simulink model "os_polyphase_synth_4096c"  (R2021a) (continued)
== system os_polyphase_synth_4096c/pfb_fir/pol1_in1_tap86 == (continued)
      | RNDSIMPLE(ACIN*B+P)
      | RNDSIMPLE(ACIN*B+P+CARRYIN)
      | RNDSIMPLE(ACIN*B+P>>17)
      | RNDSIMPLE(ACIN*B+P>>17+CARRYIN)
      | RNDSIMPLE(ACIN*B+PCIN)
      | RNDSIMPLE(ACIN*B+PCIN+CARRYIN)
      | RNDSIMPLE(ACIN*B+PCIN>>17)
      | RNDSIMPLE(ACIN*B+PCIN>>17+CARRYIN)
      | RNDSIMPLE(ACIN*BCIN)
      | RNDSIMPLE(ACIN*BCIN+C)
      | RNDSIMPLE(ACIN*BCIN+C+CARRYIN)
      | RNDSIMPLE(ACIN*BCIN+CARRYIN)
      | RNDSIMPLE(ACIN*BCIN+P)
      | RNDSIMPLE(ACIN*BCIN+P+CARRYIN)
      | RNDSIMPLE(ACIN*BCIN+P>>17)
      | RNDSIMPLE(ACIN*BCIN+P>>17+CARRYIN)
      | RNDSIMPLE(ACIN*BCIN+PCIN)
      | RNDSIMPLE(ACIN*BCIN+PCIN+CARRYIN)
      | RNDSIMPLE(ACIN*BCIN+PCIN>>17)
      | RNDSIMPLE(ACIN*BCIN+PCIN>>17+CARRYIN)
      | RNDSIMPLE(ACIN+C)
      | RNDSIMPLE(ACIN+C+CARRYIN)
      | RNDSIMPLE(ACIN+CARRYIN)
      | RNDSIMPLE(ACIN+P)
      | RNDSIMPLE(ACIN+P+CARRYIN)
      | RNDSIMPLE(ACIN+P>>17)
      | RNDSIMPLE(ACIN+P>>17+CARRYIN)
      | RNDSIMPLE(ACIN+PCIN)
      | RNDSIMPLE(ACIN+PCIN+CARRYIN)
      | RNDSIMPLE(ACIN+PCIN>>17)
      | RNDSIMPLE(ACIN+PCIN>>17+CARRYIN)
      | RNDSIMPLE(B*(A+D))
      | RNDSIMPLE(B*(A+D)+C)
      | RNDSIMPLE(B*(A+D)+C+CARRYIN)
      | RNDSIMPLE(B*(A+D)+CARRYIN)
      | RNDSIMPLE(B*(A+D)+P)
      | RNDSIMPLE(B*(A+D)+P+CARRYIN)
      | RNDSIMPLE(B*(A+D)+P>>17)
      | RNDSIMPLE(B*(A+D)+P>>17+CARRYIN)
      | RNDSIMPLE(B*(A+D)+PCIN)
      | RNDSIMPLE(B*(A+D)+PCIN+CARRYIN)
      | RNDSIMPLE(B*(A+D)+PCIN>>17)
      | RNDSIMPLE(B*(A+D)+PCIN>>17+CARRYIN)
      | RNDSIMPLE(B*(ACIN+D))
      | RNDSIMPLE(B*(ACIN+D)+C)
      | RNDSIMPLE(B*(ACIN+D)+C+CARRYIN)
      | RNDSIMPLE(B*(ACIN+D)+CARRYIN)
      | RNDSIMPLE(B*(ACIN+D)+P)
      | RNDSIMPLE(B*(ACIN+D)+P+CARRYIN)
      | RNDSIMPLE(B*(ACIN+D)+P>>17)
      | RNDSIMPLE(B*(ACIN+D)+P>>17+CARRYIN)
      | RNDSIMPLE(B*(ACIN+D)+PCIN)
      | RNDSIMPLE(B*(ACIN+D)+PCIN+CARRYIN)
      | RNDSIMPLE(B*(ACIN+D)+PCIN>>17)
      | RNDSIMPLE(B*(ACIN+D)+PCIN>>17+CARRYIN)
      | RNDSIMPLE(B*(D+A))
      | RNDSIMPLE(B*(D+A)+C)
      | RNDSIMPLE(B*(D+A)+C+CARRYIN)
      | RNDSIMPLE(B*(D+A)+CARRYIN)
      | RNDSIMPLE(B*(D+A)+P)
      | RNDSIMPLE(B*(D+A)+P+CARRYIN)
      | RNDSIMPLE(B*(D+A)+P>>17)
      | RNDSIMPLE(B*(D+A)+P>>17+CARRYIN)
      | RNDSIMPLE(B*(D+A)+PCIN)
      | RNDSIMPLE(B*(D+A)+PCIN+CARRYIN)
      | RNDSIMPLE(B*(D+A)+PCIN>>17)
      | RNDSIMPLE(B*(D+A)+PCIN>>17+CARRYIN)
      | RNDSIMPLE(B*(D+ACIN))
      | RNDSIMPLE(B*(D+ACIN)+C)
      | RNDSIMPLE(B*(D+ACIN)+C+CARRYIN)
      | RNDSIMPLE(B*(D+ACIN)+CARRYIN)
      | RNDSIMPLE(B*(D+ACIN)+P)
      | RNDSIMPLE(B*(D+ACIN)+P+CARRYIN)
      | RNDSIMPLE(B*(D+ACIN)+P>>17)
      | RNDSIMPLE(B*(D+ACIN)+P>>17+CARRYIN)
      | RNDSIMPLE(B*(D+ACIN)+PCIN)
      | RNDSIMPLE(B*(D+ACIN)+PCIN+CARRYIN)
      | RNDSIMPLE(B*(D+ACIN)+PCIN>>17)
      | RNDSIMPLE(B*(D+ACIN)+PCIN>>17+CARRYIN)
      | RNDSIMPLE(B*(D-A))
      | RNDSIMPLE(B*(D-A)+C)
      | RNDSIMPLE(B*(D-A)+C+CARRYIN)
      | RNDSIMPLE(B*(D-A)+CARRYIN)
      | RNDSIMPLE(B*(D-A)+P)
      | RNDSIMPLE(B*(D-A)+P+CARRYIN)
      | RNDSIMPLE(B*(D-A)+P>>17)
      | RNDSIMPLE(B*(D-A)+P>>17+CARRYIN)
      | RNDSIMPLE(B*(D-A)+PCIN)
      | RNDSIMPLE(B*(D-A)+PCIN+CARRYIN)
      | RNDSIMPLE(B*(D-A)+PCIN>>17)
      | RNDSIMPLE(B*(D-A)+PCIN>>17+CARRYIN)
      | RNDSIMPLE(B*(D-ACIN))
      | RNDSIMPLE(B*(D-ACIN)+C)
      | RNDSIMPLE(B*(D-ACIN)+C+CARRYIN)
      | RNDSIMPLE(B*(D-ACIN)+CARRYIN)
      | RNDSIMPLE(B*(D-ACIN)+P)
      | RNDSIMPLE(B*(D-ACIN)+P+CARRYIN)
      | RNDSIMPLE(B*(D-ACIN)+P>>17)
      | RNDSIMPLE(B*(D-ACIN)+P>>17+CARRYIN)
      | RNDSIMPLE(B*(D-ACIN)+PCIN)
      | RNDSIMPLE(B*(D-ACIN)+PCIN+CARRYIN)
      | RNDSIMPLE(B*(D-ACIN)+PCIN>>17)
      | RNDSIMPLE(B*(D-ACIN)+PCIN>>17+CARRYIN)
      | RNDSIMPLE(B*A)
      | RNDSIMPLE(B*A+C)
      | RNDSIMPLE(B*A+C+CARRYIN)
      | RNDSIMPLE(B*A+CARRYIN)
      | RNDSIMPLE(B*A+P)
      | RNDSIMPLE(B*A+P+CARRYIN)
      | RNDSIMPLE(B*A+P>>17)
      | RNDSIMPLE(B*A+P>>17+CARRYIN)
      | RNDSIMPLE(B*A+PCIN)
      | RNDSIMPLE(B*A+PCIN+CARRYIN)
      | RNDSIMPLE(B*A+PCIN>>17)
      | RNDSIMPLE(B*A+PCIN>>17+CARRYIN)
      | RNDSIMPLE(B*ACIN)
      | RNDSIMPLE(B*ACIN+C)
      | RNDSIMPLE(B*ACIN+C+CARRYIN)
      | RNDSIMPLE(B*ACIN+CARRYIN)
      | RNDSIMPLE(B*ACIN+P)
      | RNDSIMPLE(B*ACIN+P+CARRYIN)
      | RNDSIMPLE(B*ACIN+P>>17)
      | RNDSIMPLE(B*ACIN+P>>17+CARRYIN)
      | RNDSIMPLE(B*ACIN+PCIN)
      | RNDSIMPLE(B*ACIN+PCIN+CARRYIN)
      | RNDSIMPLE(B*ACIN+PCIN>>17)
      | RNDSIMPLE(B*ACIN+PCIN>>17+CARRYIN)
      | RNDSIMPLE(B*D)
      | RNDSIMPLE(B*D+C)
      | RNDSIMPLE(B*D+C+CARRYIN)
      | RNDSIMPLE(B*D+CARRYIN)
      | RNDSIMPLE(B*D+P)
      | RNDSIMPLE(B*D+P+CARRYIN)
      | RNDSIMPLE(B*D+P>>17)
      | RNDSIMPLE(B*D+P>>17+CARRYIN)
      | RNDSIMPLE(B*D+PCIN)
      | RNDSIMPLE(B*D+PCIN+CARRYIN)
      | RNDSIMPLE(B*D+PCIN>>17)
      | RNDSIMPLE(B*D+PCIN>>17+CARRYIN)
      | RNDSIMPLE(BCIN*(A+D))
      | RNDSIMPLE(BCIN*(A+D)+C)
      | RNDSIMPLE(BCIN*(A+D)+C+CARRYIN)
      | RNDSIMPLE(BCIN*(A+D)+CARRYIN)
      | RNDSIMPLE(BCIN*(A+D)+P)
      | RNDSIMPLE(BCIN*(A+D)+P+CARRYIN)
      | RNDSIMPLE(BCIN*(A+D)+P>>17)
      | RNDSIMPLE(BCIN*(A+D)+P>>17+CARRYIN)
      | RNDSIMPLE(BCIN*(A+D)+PCIN)
      | RNDSIMPLE(BCIN*(A+D)+PCIN+CARRYIN)
      | RNDSIMPLE(BCIN*(A+D)+PCIN>>17)
      | RNDSIMPLE(BCIN*(A+D)+PCIN>>17+CARRYIN)
      | RNDSIMPLE(BCIN*(ACIN+D))
      | RNDSIMPLE(BCIN*(ACIN+D)+C)
      | RNDSIMPLE(BCIN*(ACIN+D)+C+CARRYIN)
      | RNDSIMPLE(BCIN*(ACIN+D)+CARRYIN)
      | RNDSIMPLE(BCIN*(ACIN+D)+P)
      | RNDSIMPLE(BCIN*(ACIN+D)+P+CARRYIN)
      | RNDSIMPLE(BCIN*(ACIN+D)+P>>17)
      | RNDSIMPLE(BCIN*(ACIN+D)+P>>17+CARRYIN)
      | RNDSIMPLE(BCIN*(ACIN+D)+PCIN)
      | RNDSIMPLE(BCIN*(ACIN+D)+PCIN+CARRYIN)
      | RNDSIMPLE(BCIN*(ACIN+D)+PCIN>>17)
      | RNDSIMPLE(BCIN*(ACIN+D)+PCIN>>17+CARRYIN)
      | RNDSIMPLE(BCIN*(D+A))
      | RNDSIMPLE(BCIN*(D+A)+C)
      | RNDSIMPLE(BCIN*(D+A)+C+CARRYIN)
      | RNDSIMPLE(BCIN*(D+A)+CARRYIN)
      | RNDSIMPLE(BCIN*(D+A)+P)
      | RNDSIMPLE(BCIN*(D+A)+P+CARRYIN)
      | RNDSIMPLE(BCIN*(D+A)+P>>17)
      | RNDSIMPLE(BCIN*(D+A)+P>>17+CARRYIN)
      | RNDSIMPLE(BCIN*(D+A)+PCIN)
      | RNDSIMPLE(BCIN*(D+A)+PCIN+CARRYIN)
      | RNDSIMPLE(BCIN*(D+A)+PCIN>>17)
      | RNDSIMPLE(BCIN*(D+A)+PCIN>>17+CARRYIN)
      | RNDSIMPLE(BCIN*(D+ACIN))
      | RNDSIMPLE(BCIN*(D+ACIN)+C)
      | RNDSIMPLE(BCIN*(D+ACIN)+C+CARRYIN)
      | RNDSIMPLE(BCIN*(D+ACIN)+CARRYIN)
      | RNDSIMPLE(BCIN*(D+ACIN)+P)
      | RNDSIMPLE(BCIN*(D+ACIN)+P+CARRYIN)
      | RNDSIMPLE(BCIN*(D+ACIN)+P>>17)
      | RNDSIMPLE(BCIN*(D+ACIN)+P>>17+CARRYIN)
      | RNDSIMPLE(BCIN*(D+ACIN)+PCIN)
      | RNDSIMPLE(BCIN*(D+ACIN)+PCIN+CARRYIN)
      | RNDSIMPLE(BCIN*(D+ACIN)+PCIN>>17)
      | RNDSIMPLE(BCIN*(D+ACIN)+PCIN>>17+CARRYIN)
      | RNDSIMPLE(BCIN*(D-A))
      | RNDSIMPLE(BCIN*(D-A)+C)
      | RNDSIMPLE(BCIN*(D-A)+C+CARRYIN)
      | RNDSIMPLE(BCIN*(D-A)+CARRYIN)
      | RNDSIMPLE(BCIN*(D-A)+P)
      | RNDSIMPLE(BCIN*(D-A)+P+CARRYIN)
      | RNDSIMPLE(BCIN*(D-A)+P>>17)
      | RNDSIMPLE(BCIN*(D-A)+P>>17+CARRYIN)
      | RNDSIMPLE(BCIN*(D-A)+PCIN)
      | RNDSIMPLE(BCIN*(D-A)+PCIN+CARRYIN)
      | RNDSIMPLE(BCIN*(D-A)+PCIN>>17)
      | RNDSIMPLE(BCIN*(D-A)+PCIN>>17+CARRYIN)
      | RNDSIMPLE(BCIN*(D-ACIN))
      | RNDSIMPLE(BCIN*(D-ACIN)+C)
      | RNDSIMPLE(BCIN*(D-ACIN)+C+CARRYIN)
      | RNDSIMPLE(BCIN*(D-ACIN)+CARRYIN)
      | RNDSIMPLE(BCIN*(D-ACIN)+P)
      | RNDSIMPLE(BCIN*(D-ACIN)+P+CARRYIN)
      | RNDSIMPLE(BCIN*(D-ACIN)+P>>17)
      | RNDSIMPLE(BCIN*(D-ACIN)+P>>17+CARRYIN)
      | RNDSIMPLE(BCIN*(D-ACIN)+PCIN)
      | RNDSIMPLE(BCIN*(D-ACIN)+PCIN+CARRYIN)
      | RNDSIMPLE(BCIN*(D-ACIN)+PCIN>>17)
      | RNDSIMPLE(BCIN*(D-ACIN)+PCIN>>17+CARRYIN)
      | RNDSIMPLE(BCIN*A)
      | RNDSIMPLE(BCIN*A+C)
      | RNDSIMPLE(BCIN*A+C+CARRYIN)
      | RNDSIMPLE(BCIN*A+CARRYIN)
      | RNDSIMPLE(BCIN*A+P)
      | RNDSIMPLE(BCIN*A+P+CARRYIN)
      | RNDSIMPLE(BCIN*A+P>>17)
      | RNDSIMPLE(BCIN*A+P>>17+CARRYIN)
      | RNDSIMPLE(BCIN*A+PCIN)
      | RNDSIMPLE(BCIN*A+PCIN+CARRYIN)
      | RNDSIMPLE(BCIN*A+PCIN>>17)
      | RNDSIMPLE(BCIN*A+PCIN>>17+CARRYIN)
      | RNDSIMPLE(BCIN*ACIN)
      | RNDSIMPLE(BCIN*ACIN+C)
      | RNDSIMPLE(BCIN*ACIN+C+CARRYIN)
      | RNDSIMPLE(BCIN*ACIN+CARRYIN)
      | RNDSIMPLE(BCIN*ACIN+P)
      | RNDSIMPLE(BCIN*ACIN+P+CARRYIN)
      | RNDSIMPLE(BCIN*ACIN+P>>17)
      | RNDSIMPLE(BCIN*ACIN+P>>17+CARRYIN)
      | RNDSIMPLE(BCIN*ACIN+PCIN)
      | RNDSIMPLE(BCIN*ACIN+PCIN+CARRYIN)
      | RNDSIMPLE(BCIN*ACIN+PCIN>>17)
      | RNDSIMPLE(BCIN*ACIN+PCIN>>17+CARRYIN)
      | RNDSIMPLE(BCIN*D)
      | RNDSIMPLE(BCIN*D+C)
      | RNDSIMPLE(BCIN*D+C+CARRYIN)
      | RNDSIMPLE(BCIN*D+CARRYIN)
      | RNDSIMPLE(BCIN*D+P)
      | RNDSIMPLE(BCIN*D+P+CARRYIN)
      | RNDSIMPLE(BCIN*D+P>>17)
      | RNDSIMPLE(BCIN*D+P>>17+CARRYIN)
      | RNDSIMPLE(BCIN*D+PCIN)
      | RNDSIMPLE(BCIN*D+PCIN+CARRYIN)
      | RNDSIMPLE(BCIN*D+PCIN>>17)
      | RNDSIMPLE(BCIN*D+PCIN>>17+CARRYIN)
      | RNDSIMPLE(C)
      | RNDSIMPLE(C+(A+D))
      | RNDSIMPLE(C+(A+D)*(A+D))
      | RNDSIMPLE(C+(A+D)*(A+D)+CARRYIN)
      | RNDSIMPLE(C+(A+D)*B)
      | RNDSIMPLE(C+(A+D)*B+CARRYIN)
      | RNDSIMPLE(C+(A+D)*BCIN)
      | RNDSIMPLE(C+(A+D)*BCIN+CARRYIN)
      | RNDSIMPLE(C+(A+D)+CARRYIN)
      | RNDSIMPLE(C+(ACIN+D))
      | RNDSIMPLE(C+(ACIN+D)*(ACIN+D))
      | RNDSIMPLE(C+(ACIN+D)*(ACIN+D)+CARRYIN)
      | RNDSIMPLE(C+(ACIN+D)*B)
      | RNDSIMPLE(C+(ACIN+D)*B+CARRYIN)
      | RNDSIMPLE(C+(ACIN+D)*BCIN)
      | RNDSIMPLE(C+(ACIN+D)*BCIN+CARRYIN)
      | RNDSIMPLE(C+(ACIN+D)+CARRYIN)
      | RNDSIMPLE(C+(D+A))
      | RNDSIMPLE(C+(D+A)*(D+A))
      | RNDSIMPLE(C+(D+A)*(D+A)+CARRYIN)
      | RNDSIMPLE(C+(D+A)*B)
      | RNDSIMPLE(C+(D+A)*B+CARRYIN)
      | RNDSIMPLE(C+(D+A)*BCIN)
      | RNDSIMPLE(C+(D+A)*BCIN+CARRYIN)
      | RNDSIMPLE(C+(D+A)+CARRYIN)
      | RNDSIMPLE(C+(D+ACIN))
      | RNDSIMPLE(C+(D+ACIN)*(D+ACIN))
      | RNDSIMPLE(C+(D+ACIN)*(D+ACIN)+CARRYIN)
      | RNDSIMPLE(C+(D+ACIN)*B)
      | RNDSIMPLE(C+(D+ACIN)*B+CARRYIN)
      | RNDSIMPLE(C+(D+ACIN)*BCIN)
      | RNDSIMPLE(C+(D+ACIN)*BCIN+CARRYIN)
      | RNDSIMPLE(C+(D+ACIN)+CARRYIN)
      | RNDSIMPLE(C+(D-A))
      | RNDSIMPLE(C+(D-A)*(D-A))
      | RNDSIMPLE(C+(D-A)*(D-A)+CARRYIN)
      | RNDSIMPLE(C+(D-A)*B)
      | RNDSIMPLE(C+(D-A)*B+CARRYIN)
      | RNDSIMPLE(C+(D-A)*BCIN)
      | RNDSIMPLE(C+(D-A)*BCIN+CARRYIN)
      | RNDSIMPLE(C+(D-A)+CARRYIN)
      | RNDSIMPLE(C+(D-ACIN))
      | RNDSIMPLE(C+(D-ACIN)*(D-ACIN))
      | RNDSIMPLE(C+(D-ACIN)*(D-ACIN)+CARRYIN)
      | RNDSIMPLE(C+(D-ACIN)*B)
      | RNDSIMPLE(C+(D-ACIN)*B+CARRYIN)
      | RNDSIMPLE(C+(D-ACIN)*BCIN)
      | RNDSIMPLE(C+(D-ACIN)*BCIN+CARRYIN)
      | RNDSIMPLE(C+(D-ACIN)+CARRYIN)
      | RNDSIMPLE(C+A)
      | RNDSIMPLE(C+A*A)
      | RNDSIMPLE(C+A*A+CARRYIN)
      | RNDSIMPLE(C+A*B)
      | RNDSIMPLE(C+A*B+CARRYIN)
      | RNDSIMPLE(C+A*BCIN)
      | RNDSIMPLE(C+A*BCIN+CARRYIN)
      | RNDSIMPLE(C+A+CARRYIN)
      | RNDSIMPLE(C+ACIN)
      | RNDSIMPLE(C+ACIN*ACIN)
      | RNDSIMPLE(C+ACIN*ACIN+CARRYIN)
      | RNDSIMPLE(C+ACIN*B)
      | RNDSIMPLE(C+ACIN*B+CARRYIN)
      | RNDSIMPLE(C+ACIN*BCIN)
      | RNDSIMPLE(C+ACIN*BCIN+CARRYIN)
      | RNDSIMPLE(C+ACIN+CARRYIN)
      | RNDSIMPLE(C+B*(A+D))
      | RNDSIMPLE(C+B*(A+D)+CARRYIN)
      | RNDSIMPLE(C+B*(ACIN+D))
      | RNDSIMPLE(C+B*(ACIN+D)+CARRYIN)
      | RNDSIMPLE(C+B*(D+A))
      | RNDSIMPLE(C+B*(D+A)+CARRYIN)
      | RNDSIMPLE(C+B*(D+ACIN))
      | RNDSIMPLE(C+B*(D+ACIN)+CARRYIN)
      | RNDSIMPLE(C+B*(D-A))
      | RNDSIMPLE(C+B*(D-A)+CARRYIN)
      | RNDSIMPLE(C+B*(D-ACIN))
      | RNDSIMPLE(C+B*(D-ACIN)+CARRYIN)
      | RNDSIMPLE(C+B*A)
      | RNDSIMPLE(C+B*A+CARRYIN)
      | RNDSIMPLE(C+B*ACIN)
      | RNDSIMPLE(C+B*ACIN+CARRYIN)
      | RNDSIMPLE(C+B*D)
      | RNDSIMPLE(C+B*D+CARRYIN)
      | RNDSIMPLE(C+BCIN*(A+D))
      | RNDSIMPLE(C+BCIN*(A+D)+CARRYIN)
      | RNDSIMPLE(C+BCIN*(ACIN+D))
      | RNDSIMPLE(C+BCIN*(ACIN+D)+CARRYIN)
      | RNDSIMPLE(C+BCIN*(D+A))
      | RNDSIMPLE(C+BCIN*(D+A)+CARRYIN)
      | RNDSIMPLE(C+BCIN*(D+ACIN))
      | RNDSIMPLE(C+BCIN*(D+ACIN)+CARRYIN)
      | RNDSIMPLE(C+BCIN*(D-A))
      | RNDSIMPLE(C+BCIN*(D-A)+CARRYIN)
      | RNDSIMPLE(C+BCIN*(D-ACIN))
      | RNDSIMPLE(C+BCIN*(D-ACIN)+CARRYIN)
      | RNDSIMPLE(C+BCIN*A)
      | RNDSIMPLE(C+BCIN*A+CARRYIN)
      | RNDSIMPLE(C+BCIN*ACIN)
      | RNDSIMPLE(C+BCIN*ACIN+CARRYIN)
      | RNDSIMPLE(C+BCIN*D)
      | RNDSIMPLE(C+BCIN*D+CARRYIN)
      | RNDSIMPLE(C+C)
      | RNDSIMPLE(C+C+CARRYIN)
      | RNDSIMPLE(C+CARRYCASCIN)
      | RNDSIMPLE(C+CARRYIN)
      | RNDSIMPLE(C+CONCAT)
      | RNDSIMPLE(C+CONCAT+C)
      | RNDSIMPLE(C+CONCAT+C+CARRYIN)
      | RNDSIMPLE(C+CONCAT+CARRYIN)
      | RNDSIMPLE(C+D)
      | RNDSIMPLE(C+D*B)
      | RNDSIMPLE(C+D*B+CARRYIN)
      | RNDSIMPLE(C+D*BCIN)
      | RNDSIMPLE(C+D*BCIN+CARRYIN)
      | RNDSIMPLE(C+D*D)
      | RNDSIMPLE(C+D*D+CARRYIN)
      | RNDSIMPLE(C+D+CARRYIN)
      | RNDSIMPLE(C+P)
      | RNDSIMPLE(C+P+C)
      | RNDSIMPLE(C+P+C+CARRYIN)
      | RNDSIMPLE(C+P+CARRYIN)
      | RNDSIMPLE(CARRYIN)
      | RNDSIMPLE(CONCAT)
      | RNDSIMPLE(CONCAT+C)
      | RNDSIMPLE(CONCAT+C+CARRYIN)
      | RNDSIMPLE(CONCAT+CARRYCASCIN)
      | RNDSIMPLE(CONCAT+CARRYIN)
      | RNDSIMPLE(D)
      | RNDSIMPLE(D*B)
      | RNDSIMPLE(D*B+C)
      | RNDSIMPLE(D*B+C+CARRYIN)
      | RNDSIMPLE(D*B+CARRYIN)
      | RNDSIMPLE(D*B+P)
      | RNDSIMPLE(D*B+P+CARRYIN)
      | RNDSIMPLE(D*B+P>>17)
      | RNDSIMPLE(D*B+P>>17+CARRYIN)
      | RNDSIMPLE(D*B+PCIN)
      | RNDSIMPLE(D*B+PCIN+CARRYIN)
      | RNDSIMPLE(D*B+PCIN>>17)
      | RNDSIMPLE(D*B+PCIN>>17+CARRYIN)
      | RNDSIMPLE(D*BCIN)
      | RNDSIMPLE(D*BCIN+C)
      | RNDSIMPLE(D*BCIN+C+CARRYIN)
      | RNDSIMPLE(D*BCIN+CARRYIN)
      | RNDSIMPLE(D*BCIN+P)
      | RNDSIMPLE(D*BCIN+P+CARRYIN)
      | RNDSIMPLE(D*BCIN+P>>17)
      | RNDSIMPLE(D*BCIN+P>>17+CARRYIN)
      | RNDSIMPLE(D*BCIN+PCIN)
      | RNDSIMPLE(D*BCIN+PCIN+CARRYIN)
      | RNDSIMPLE(D*BCIN+PCIN>>17)
      | RNDSIMPLE(D*BCIN+PCIN>>17+CARRYIN)
      | RNDSIMPLE(D*D)
      | RNDSIMPLE(D*D+C)
      | RNDSIMPLE(D*D+C+CARRYIN)
      | RNDSIMPLE(D*D+CARRYIN)
      | RNDSIMPLE(D*D+P)
      | RNDSIMPLE(D*D+P+CARRYIN)
      | RNDSIMPLE(D*D+P>>17)
      | RNDSIMPLE(D*D+P>>17+CARRYIN)
      | RNDSIMPLE(D*D+PCIN)
      | RNDSIMPLE(D*D+PCIN+CARRYIN)
      | RNDSIMPLE(D*D+PCIN>>17)
      | RNDSIMPLE(D*D+PCIN>>17+CARRYIN)
      | RNDSIMPLE(D+C)
      | RNDSIMPLE(D+C+CARRYIN)
      | RNDSIMPLE(D+CARRYIN)
      | RNDSIMPLE(D+P)
      | RNDSIMPLE(D+P+CARRYIN)
      | RNDSIMPLE(D+P>>17)
      | RNDSIMPLE(D+P>>17+CARRYIN)
      | RNDSIMPLE(D+PCIN)
      | RNDSIMPLE(D+PCIN+CARRYIN)
      | RNDSIMPLE(D+PCIN>>17)
      | RNDSIMPLE(D+PCIN>>17+CARRYIN)
      | RNDSIMPLE(P)
      | RNDSIMPLE(P+(A+D))
      | RNDSIMPLE(P+(A+D)*(A+D))
      | RNDSIMPLE(P+(A+D)*(A+D)+CARRYIN)
      | RNDSIMPLE(P+(A+D)*B)
      | RNDSIMPLE(P+(A+D)*B+CARRYIN)
      | RNDSIMPLE(P+(A+D)*BCIN)
      | RNDSIMPLE(P+(A+D)*BCIN+CARRYIN)
      | RNDSIMPLE(P+(A+D)+CARRYIN)
      | RNDSIMPLE(P+(ACIN+D))
      | RNDSIMPLE(P+(ACIN+D)*(ACIN+D))
      | RNDSIMPLE(P+(ACIN+D)*(ACIN+D)+CARRYIN)
      | RNDSIMPLE(P+(ACIN+D)*B)
      | RNDSIMPLE(P+(ACIN+D)*B+CARRYIN)
      | RNDSIMPLE(P+(ACIN+D)*BCIN)
      | RNDSIMPLE(P+(ACIN+D)*BCIN+CARRYIN)
      | RNDSIMPLE(P+(ACIN+D)+CARRYIN)
      | RNDSIMPLE(P+(D+A))
      | RNDSIMPLE(P+(D+A)*(D+A))
      | RNDSIMPLE(P+(D+A)*(D+A)+CARRYIN)
      | RNDSIMPLE(P+(D+A)*B)
      | RNDSIMPLE(P+(D+A)*B+CARRYIN)
      | RNDSIMPLE(P+(D+A)*BCIN)
      | RNDSIMPLE(P+(D+A)*BCIN+CARRYIN)
      | RNDSIMPLE(P+(D+A)+CARRYIN)
      | RNDSIMPLE(P+(D+ACIN))
      | RNDSIMPLE(P+(D+ACIN)*(D+ACIN))
      | RNDSIMPLE(P+(D+ACIN)*(D+ACIN)+CARRYIN)
      | RNDSIMPLE(P+(D+ACIN)*B)
      | RNDSIMPLE(P+(D+ACIN)*B+CARRYIN)
      | RNDSIMPLE(P+(D+ACIN)*BCIN)
      | RNDSIMPLE(P+(D+ACIN)*BCIN+CARRYIN)
      | RNDSIMPLE(P+(D+ACIN)+CARRYIN)
      | RNDSIMPLE(P+(D-A))
      | RNDSIMPLE(P+(D-A)*(D-A))
      | RNDSIMPLE(P+(D-A)*(D-A)+CARRYIN)
      | RNDSIMPLE(P+(D-A)*B)
      | RNDSIMPLE(P+(D-A)*B+CARRYIN)
      | RNDSIMPLE(P+(D-A)*BCIN)
      | RNDSIMPLE(P+(D-A)*BCIN+CARRYIN)
      | RNDSIMPLE(P+(D-A)+CARRYIN)
      | RNDSIMPLE(P+(D-ACIN))
      | RNDSIMPLE(P+(D-ACIN)*(D-ACIN))
      | RNDSIMPLE(P+(D-ACIN)*(D-ACIN)+CARRYIN)
      | RNDSIMPLE(P+(D-ACIN)*B)
      | RNDSIMPLE(P+(D-ACIN)*B+CARRYIN)
      | RNDSIMPLE(P+(D-ACIN)*BCIN)
      | RNDSIMPLE(P+(D-ACIN)*BCIN+CARRYIN)
      | RNDSIMPLE(P+(D-ACIN)+CARRYIN)
      | RNDSIMPLE(P+A)
      | RNDSIMPLE(P+A*A)
      | RNDSIMPLE(P+A*A+CARRYIN)
      | RNDSIMPLE(P+A*B)
      | RNDSIMPLE(P+A*B+CARRYIN)
      | RNDSIMPLE(P+A*BCIN)
      | RNDSIMPLE(P+A*BCIN+CARRYIN)
      | RNDSIMPLE(P+A+CARRYIN)
      | RNDSIMPLE(P+ACIN)
      | RNDSIMPLE(P+ACIN*ACIN)
      | RNDSIMPLE(P+ACIN*ACIN+CARRYIN)
      | RNDSIMPLE(P+ACIN*B)
      | RNDSIMPLE(P+ACIN*B+CARRYIN)
      | RNDSIMPLE(P+ACIN*BCIN)
      | RNDSIMPLE(P+ACIN*BCIN+CARRYIN)
      | RNDSIMPLE(P+ACIN+CARRYIN)
      | RNDSIMPLE(P+B*(A+D))
      | RNDSIMPLE(P+B*(A+D)+CARRYIN)
      | RNDSIMPLE(P+B*(ACIN+D))
      | RNDSIMPLE(P+B*(ACIN+D)+CARRYIN)
      | RNDSIMPLE(P+B*(D+A))
      | RNDSIMPLE(P+B*(D+A)+CARRYIN)
      | RNDSIMPLE(P+B*(D+ACIN))
      | RNDSIMPLE(P+B*(D+ACIN)+CARRYIN)
      | RNDSIMPLE(P+B*(D-A))
      | RNDSIMPLE(P+B*(D-A)+CARRYIN)
      | RNDSIMPLE(P+B*(D-ACIN))
      | RNDSIMPLE(P+B*(D-ACIN)+CARRYIN)
      | RNDSIMPLE(P+B*A)
      | RNDSIMPLE(P+B*A+CARRYIN)
      | RNDSIMPLE(P+B*ACIN)
      | RNDSIMPLE(P+B*ACIN+CARRYIN)
      | RNDSIMPLE(P+B*D)
      | RNDSIMPLE(P+B*D+CARRYIN)
      | RNDSIMPLE(P+BCIN*(A+D))
      | RNDSIMPLE(P+BCIN*(A+D)+CARRYIN)
      | RNDSIMPLE(P+BCIN*(ACIN+D))
      | RNDSIMPLE(P+BCIN*(ACIN+D)+CARRYIN)
      | RNDSIMPLE(P+BCIN*(D+A))
      | RNDSIMPLE(P+BCIN*(D+A)+CARRYIN)
      | RNDSIMPLE(P+BCIN*(D+ACIN))
      | RNDSIMPLE(P+BCIN*(D+ACIN)+CARRYIN)
      | RNDSIMPLE(P+BCIN*(D-A))
      | RNDSIMPLE(P+BCIN*(D-A)+CARRYIN)
      | RNDSIMPLE(P+BCIN*(D-ACIN))
      | RNDSIMPLE(P+BCIN*(D-ACIN)+CARRYIN)
      | RNDSIMPLE(P+BCIN*A)
      | RNDSIMPLE(P+BCIN*A+CARRYIN)
      | RNDSIMPLE(P+BCIN*ACIN)
      | RNDSIMPLE(P+BCIN*ACIN+CARRYIN)
      | RNDSIMPLE(P+BCIN*D)
      | RNDSIMPLE(P+BCIN*D+CARRYIN)
      | RNDSIMPLE(P+C)
      | RNDSIMPLE(P+C+CARRYIN)
      | RNDSIMPLE(P+CARRYCASCIN)
      | RNDSIMPLE(P+CARRYIN)
      | RNDSIMPLE(P+CONCAT)
      | RNDSIMPLE(P+CONCAT+C)
      | RNDSIMPLE(P+CONCAT+C+CARRYIN)
      | RNDSIMPLE(P+CONCAT+CARRYIN)
      | RNDSIMPLE(P+D)
      | RNDSIMPLE(P+D*B)
      | RNDSIMPLE(P+D*B+CARRYIN)
      | RNDSIMPLE(P+D*BCIN)
      | RNDSIMPLE(P+D*BCIN+CARRYIN)
      | RNDSIMPLE(P+D*D)
      | RNDSIMPLE(P+D*D+CARRYIN)
      | RNDSIMPLE(P+D+CARRYIN)
      | RNDSIMPLE(P+P)
      | RNDSIMPLE(P+P+C)
      | RNDSIMPLE(P+P+C+CARRYIN)
      | RNDSIMPLE(P+P+CARRYIN)
      | RNDSIMPLE(P>>17)
      | RNDSIMPLE(P>>17+(A+D))
      | RNDSIMPLE(P>>17+(A+D)*(A+D))
      | RNDSIMPLE(P>>17+(A+D)*(A+D)+CARRYIN)
      | RNDSIMPLE(P>>17+(A+D)*B)
      | RNDSIMPLE(P>>17+(A+D)*B+CARRYIN)
      | RNDSIMPLE(P>>17+(A+D)*BCIN)
      | RNDSIMPLE(P>>17+(A+D)*BCIN+CARRYIN)
      | RNDSIMPLE(P>>17+(A+D)+CARRYIN)
      | RNDSIMPLE(P>>17+(ACIN+D))
      | RNDSIMPLE(P>>17+(ACIN+D)*(ACIN+D))
      | RNDSIMPLE(P>>17+(ACIN+D)*(ACIN+D)+CARRYIN)
      | RNDSIMPLE(P>>17+(ACIN+D)*B)
      | RNDSIMPLE(P>>17+(ACIN+D)*B+CARRYIN)
      | RNDSIMPLE(P>>17+(ACIN+D)*BCIN)
      | RNDSIMPLE(P>>17+(ACIN+D)*BCIN+CARRYIN)
      | RNDSIMPLE(P>>17+(ACIN+D)+CARRYIN)
      | RNDSIMPLE(P>>17+(D+A))
      | RNDSIMPLE(P>>17+(D+A)*(D+A))
      | RNDSIMPLE(P>>17+(D+A)*(D+A)+CARRYIN)
      | RNDSIMPLE(P>>17+(D+A)*B)
      | RNDSIMPLE(P>>17+(D+A)*B+CARRYIN)
      | RNDSIMPLE(P>>17+(D+A)*BCIN)
      | RNDSIMPLE(P>>17+(D+A)*BCIN+CARRYIN)
      | RNDSIMPLE(P>>17+(D+A)+CARRYIN)
      | RNDSIMPLE(P>>17+(D+ACIN))
      | RNDSIMPLE(P>>17+(D+ACIN)*(D+ACIN))
      | RNDSIMPLE(P>>17+(D+ACIN)*(D+ACIN)+CARRYIN)
      | RNDSIMPLE(P>>17+(D+ACIN)*B)
      | RNDSIMPLE(P>>17+(D+ACIN)*B+CARRYIN)
      | RNDSIMPLE(P>>17+(D+ACIN)*BCIN)
      | RNDSIMPLE(P>>17+(D+ACIN)*BCIN+CARRYIN)
      | RNDSIMPLE(P>>17+(D+ACIN)+CARRYIN)
      | RNDSIMPLE(P>>17+(D-A))
      | RNDSIMPLE(P>>17+(D-A)*(D-A))
      | RNDSIMPLE(P>>17+(D-A)*(D-A)+CARRYIN)
      | RNDSIMPLE(P>>17+(D-A)*B)
      | RNDSIMPLE(P>>17+(D-A)*B+CARRYIN)
      | RNDSIMPLE(P>>17+(D-A)*BCIN)
      | RNDSIMPLE(P>>17+(D-A)*BCIN+CARRYIN)
      | RNDSIMPLE(P>>17+(D-A)+CARRYIN)
      | RNDSIMPLE(P>>17+(D-ACIN))
      | RNDSIMPLE(P>>17+(D-ACIN)*(D-ACIN))
      | RNDSIMPLE(P>>17+(D-ACIN)*(D-ACIN)+CARRYIN)
      | RNDSIMPLE(P>>17+(D-ACIN)*B)
      | RNDSIMPLE(P>>17+(D-ACIN)*B+CARRYIN)
      | RNDSIMPLE(P>>17+(D-ACIN)*BCIN)
      | RNDSIMPLE(P>>17+(D-ACIN)*BCIN+CARRYIN)
      | RNDSIMPLE(P>>17+(D-ACIN)+CARRYIN)
      | RNDSIMPLE(P>>17+A)
      | RNDSIMPLE(P>>17+A*A)
      | RNDSIMPLE(P>>17+A*A+CARRYIN)
      | RNDSIMPLE(P>>17+A*B)
      | RNDSIMPLE(P>>17+A*B+CARRYIN)
      | RNDSIMPLE(P>>17+A*BCIN)
      | RNDSIMPLE(P>>17+A*BCIN+CARRYIN)
      | RNDSIMPLE(P>>17+A+CARRYIN)
      | RNDSIMPLE(P>>17+ACIN)
      | RNDSIMPLE(P>>17+ACIN*ACIN)
      | RNDSIMPLE(P>>17+ACIN*ACIN+CARRYIN)
      | RNDSIMPLE(P>>17+ACIN*B)
      | RNDSIMPLE(P>>17+ACIN*B+CARRYIN)
      | RNDSIMPLE(P>>17+ACIN*BCIN)
      | RNDSIMPLE(P>>17+ACIN*BCIN+CARRYIN)
      | RNDSIMPLE(P>>17+ACIN+CARRYIN)
      | RNDSIMPLE(P>>17+B*(A+D))
      | RNDSIMPLE(P>>17+B*(A+D)+CARRYIN)
      | RNDSIMPLE(P>>17+B*(ACIN+D))
      | RNDSIMPLE(P>>17+B*(ACIN+D)+CARRYIN)
      | RNDSIMPLE(P>>17+B*(D+A))
      | RNDSIMPLE(P>>17+B*(D+A)+CARRYIN)
      | RNDSIMPLE(P>>17+B*(D+ACIN))
      | RNDSIMPLE(P>>17+B*(D+ACIN)+CARRYIN)
      | RNDSIMPLE(P>>17+B*(D-A))
      | RNDSIMPLE(P>>17+B*(D-A)+CARRYIN)
      | RNDSIMPLE(P>>17+B*(D-ACIN))
      | RNDSIMPLE(P>>17+B*(D-ACIN)+CARRYIN)
      | RNDSIMPLE(P>>17+B*A)
      | RNDSIMPLE(P>>17+B*A+CARRYIN)
      | RNDSIMPLE(P>>17+B*ACIN)
      | RNDSIMPLE(P>>17+B*ACIN+CARRYIN)
      | RNDSIMPLE(P>>17+B*D)
      | RNDSIMPLE(P>>17+B*D+CARRYIN)
      | RNDSIMPLE(P>>17+BCIN*(A+D))
      | RNDSIMPLE(P>>17+BCIN*(A+D)+CARRYIN)
      | RNDSIMPLE(P>>17+BCIN*(ACIN+D))
      | RNDSIMPLE(P>>17+BCIN*(ACIN+D)+CARRYIN)
      | RNDSIMPLE(P>>17+BCIN*(D+A))
      | RNDSIMPLE(P>>17+BCIN*(D+A)+CARRYIN)
      | RNDSIMPLE(P>>17+BCIN*(D+ACIN))
      | RNDSIMPLE(P>>17+BCIN*(D+ACIN)+CARRYIN)
      | RNDSIMPLE(P>>17+BCIN*(D-A))
      | RNDSIMPLE(P>>17+BCIN*(D-A)+CARRYIN)
      | RNDSIMPLE(P>>17+BCIN*(D-ACIN))
      | RNDSIMPLE(P>>17+BCIN*(D-ACIN)+CARRYIN)
      | RNDSIMPLE(P>>17+BCIN*A)
      | RNDSIMPLE(P>>17+BCIN*A+CARRYIN)
      | RNDSIMPLE(P>>17+BCIN*ACIN)
      | RNDSIMPLE(P>>17+BCIN*ACIN+CARRYIN)
      | RNDSIMPLE(P>>17+BCIN*D)
      | RNDSIMPLE(P>>17+BCIN*D+CARRYIN)
      | RNDSIMPLE(P>>17+C)
      | RNDSIMPLE(P>>17+C+CARRYIN)
      | RNDSIMPLE(P>>17+CARRYIN)
      | RNDSIMPLE(P>>17+CONCAT)
      | RNDSIMPLE(P>>17+CONCAT+C)
      | RNDSIMPLE(P>>17+CONCAT+C+CARRYIN)
      | RNDSIMPLE(P>>17+CONCAT+CARRYIN)
      | RNDSIMPLE(P>>17+D)
      | RNDSIMPLE(P>>17+D*B)
      | RNDSIMPLE(P>>17+D*B+CARRYIN)
      | RNDSIMPLE(P>>17+D*BCIN)
      | RNDSIMPLE(P>>17+D*BCIN+CARRYIN)
      | RNDSIMPLE(P>>17+D*D)
      | RNDSIMPLE(P>>17+D*D+CARRYIN)
      | RNDSIMPLE(P>>17+D+CARRYIN)
      | RNDSIMPLE(P>>17+P)
      | RNDSIMPLE(P>>17+P+C)
      | RNDSIMPLE(P>>17+P+C+CARRYIN)
      | RNDSIMPLE(P>>17+P+CARRYIN)
      | RNDSIMPLE(PCIN)
      | RNDSIMPLE(PCIN+(A+D))
      | RNDSIMPLE(PCIN+(A+D)*(A+D))
      | RNDSIMPLE(PCIN+(A+D)*(A+D)+CARRYIN)
      | RNDSIMPLE(PCIN+(A+D)*B)
      | RNDSIMPLE(PCIN+(A+D)*B+CARRYIN)
      | RNDSIMPLE(PCIN+(A+D)*BCIN)
      | RNDSIMPLE(PCIN+(A+D)*BCIN+CARRYIN)
      | RNDSIMPLE(PCIN+(A+D)+CARRYIN)
      | RNDSIMPLE(PCIN+(ACIN+D))
      | RNDSIMPLE(PCIN+(ACIN+D)*(ACIN+D))
      | RNDSIMPLE(PCIN+(ACIN+D)*(ACIN+D)+CARRYIN)
      | RNDSIMPLE(PCIN+(ACIN+D)*B)
      | RNDSIMPLE(PCIN+(ACIN+D)*B+CARRYIN)
      | RNDSIMPLE(PCIN+(ACIN+D)*BCIN)
      | RNDSIMPLE(PCIN+(ACIN+D)*BCIN+CARRYIN)
      | RNDSIMPLE(PCIN+(ACIN+D)+CARRYIN)
      | RNDSIMPLE(PCIN+(D+A))
      | RNDSIMPLE(PCIN+(D+A)*(D+A))
      | RNDSIMPLE(PCIN+(D+A)*(D+A)+CARRYIN)
      | RNDSIMPLE(PCIN+(D+A)*B)
      | RNDSIMPLE(PCIN+(D+A)*B+CARRYIN)
      | RNDSIMPLE(PCIN+(D+A)*BCIN)
      | RNDSIMPLE(PCIN+(D+A)*BCIN+CARRYIN)
      | RNDSIMPLE(PCIN+(D+A)+CARRYIN)
      | RNDSIMPLE(PCIN+(D+ACIN))
      | RNDSIMPLE(PCIN+(D+ACIN)*(D+ACIN))
      | RNDSIMPLE(PCIN+(D+ACIN)*(D+ACIN)+CARRYIN)
      | RNDSIMPLE(PCIN+(D+ACIN)*B)
      | RNDSIMPLE(PCIN+(D+ACIN)*B+CARRYIN)
      | RNDSIMPLE(PCIN+(D+ACIN)*BCIN)
      | RNDSIMPLE(PCIN+(D+ACIN)*BCIN+CARRYIN)
      | RNDSIMPLE(PCIN+(D+ACIN)+CARRYIN)
      | RNDSIMPLE(PCIN+(D-A))
      | RNDSIMPLE(PCIN+(D-A)*(D-A))
      | RNDSIMPLE(PCIN+(D-A)*(D-A)+CARRYIN)
      | RNDSIMPLE(PCIN+(D-A)*B)
      | RNDSIMPLE(PCIN+(D-A)*B+CARRYIN)
      | RNDSIMPLE(PCIN+(D-A)*BCIN)
      | RNDSIMPLE(PCIN+(D-A)*BCIN+CARRYIN)
      | RNDSIMPLE(PCIN+(D-A)+CARRYIN)
      | RNDSIMPLE(PCIN+(D-ACIN))
      | RNDSIMPLE(PCIN+(D-ACIN)*(D-ACIN))
      | RNDSIMPLE(PCIN+(D-ACIN)*(D-ACIN)+CARRYIN)
      | RNDSIMPLE(PCIN+(D-ACIN)*B)
      | RNDSIMPLE(PCIN+(D-ACIN)*B+CARRYIN)
      | RNDSIMPLE(PCIN+(D-ACIN)*BCIN)
      | RNDSIMPLE(PCIN+(D-ACIN)*BCIN+CARRYIN)
      | RNDSIMPLE(PCIN+(D-ACIN)+CARRYIN)
      | RNDSIMPLE(PCIN+A)
      | RNDSIMPLE(PCIN+A*A)
      | RNDSIMPLE(PCIN+A*A+CARRYIN)
      | RNDSIMPLE(PCIN+A*B)
      | RNDSIMPLE(PCIN+A*B+CARRYIN)
      | RNDSIMPLE(PCIN+A*BCIN)
      | RNDSIMPLE(PCIN+A*BCIN+CARRYIN)
      | RNDSIMPLE(PCIN+A+CARRYIN)
      | RNDSIMPLE(PCIN+ACIN)
      | RNDSIMPLE(PCIN+ACIN*ACIN)
      | RNDSIMPLE(PCIN+ACIN*ACIN+CARRYIN)
      | RNDSIMPLE(PCIN+ACIN*B)
      | RNDSIMPLE(PCIN+ACIN*B+CARRYIN)
      | RNDSIMPLE(PCIN+ACIN*BCIN)
      | RNDSIMPLE(PCIN+ACIN*BCIN+CARRYIN)
      | RNDSIMPLE(PCIN+ACIN+CARRYIN)
      | RNDSIMPLE(PCIN+B*(A+D))
      | RNDSIMPLE(PCIN+B*(A+D)+CARRYIN)
      | RNDSIMPLE(PCIN+B*(ACIN+D))
      | RNDSIMPLE(PCIN+B*(ACIN+D)+CARRYIN)
      | RNDSIMPLE(PCIN+B*(D+A))
      | RNDSIMPLE(PCIN+B*(D+A)+CARRYIN)
      | RNDSIMPLE(PCIN+B*(D+ACIN))
      | RNDSIMPLE(PCIN+B*(D+ACIN)+CARRYIN)
      | RNDSIMPLE(PCIN+B*(D-A))
      | RNDSIMPLE(PCIN+B*(D-A)+CARRYIN)
      | RNDSIMPLE(PCIN+B*(D-ACIN))
      | RNDSIMPLE(PCIN+B*(D-ACIN)+CARRYIN)
      | RNDSIMPLE(PCIN+B*A)
      | RNDSIMPLE(PCIN+B*A+CARRYIN)
      | RNDSIMPLE(PCIN+B*ACIN)
      | RNDSIMPLE(PCIN+B*ACIN+CARRYIN)
      | RNDSIMPLE(PCIN+B*D)
      | RNDSIMPLE(PCIN+B*D+CARRYIN)
      | RNDSIMPLE(PCIN+BCIN*(A+D))
      | RNDSIMPLE(PCIN+BCIN*(A+D)+CARRYIN)
      | RNDSIMPLE(PCIN+BCIN*(ACIN+D))
      | RNDSIMPLE(PCIN+BCIN*(ACIN+D)+CARRYIN)
      | RNDSIMPLE(PCIN+BCIN*(D+A))
      | RNDSIMPLE(PCIN+BCIN*(D+A)+CARRYIN)
      | RNDSIMPLE(PCIN+BCIN*(D+ACIN))
      | RNDSIMPLE(PCIN+BCIN*(D+ACIN)+CARRYIN)
      | RNDSIMPLE(PCIN+BCIN*(D-A))
      | RNDSIMPLE(PCIN+BCIN*(D-A)+CARRYIN)
      | RNDSIMPLE(PCIN+BCIN*(D-ACIN))
      | RNDSIMPLE(PCIN+BCIN*(D-ACIN)+CARRYIN)
      | RNDSIMPLE(PCIN+BCIN*A)
      | RNDSIMPLE(PCIN+BCIN*A+CARRYIN)
      | RNDSIMPLE(PCIN+BCIN*ACIN)
      | RNDSIMPLE(PCIN+BCIN*ACIN+CARRYIN)
      | RNDSIMPLE(PCIN+BCIN*D)
      | RNDSIMPLE(PCIN+BCIN*D+CARRYIN)
      | RNDSIMPLE(PCIN+C)
      | RNDSIMPLE(PCIN+C+CARRYIN)
      | RNDSIMPLE(PCIN+CARRYIN)
      | RNDSIMPLE(PCIN+CONCAT)
      | RNDSIMPLE(PCIN+CONCAT+C)
      | RNDSIMPLE(PCIN+CONCAT+C+CARRYIN)
      | RNDSIMPLE(PCIN+CONCAT+CARRYIN)
      | RNDSIMPLE(PCIN+D)
      | RNDSIMPLE(PCIN+D*B)
      | RNDSIMPLE(PCIN+D*B+CARRYIN)
      | RNDSIMPLE(PCIN+D*BCIN)
      | RNDSIMPLE(PCIN+D*BCIN+CARRYIN)
      | RNDSIMPLE(PCIN+D*D)
      | RNDSIMPLE(PCIN+D*D+CARRYIN)
      | RNDSIMPLE(PCIN+D+CARRYIN)
      | RNDSIMPLE(PCIN+P)
      | RNDSIMPLE(PCIN+P+C)
      | RNDSIMPLE(PCIN+P+C+CARRYIN)
      | RNDSIMPLE(PCIN+P+CARRYIN)
      | RNDSIMPLE(PCIN>>17)
      | RNDSIMPLE(PCIN>>17+(A+D))
      | RNDSIMPLE(PCIN>>17+(A+D)*(A+D))
      | RNDSIMPLE(PCIN>>17+(A+D)*(A+D)+CARRYIN)
      | RNDSIMPLE(PCIN>>17+(A+D)*B)
      | RNDSIMPLE(PCIN>>17+(A+D)*B+CARRYIN)
      | RNDSIMPLE(PCIN>>17+(A+D)*BCIN)
      | RNDSIMPLE(PCIN>>17+(A+D)*BCIN+CARRYIN)
      | RNDSIMPLE(PCIN>>17+(A+D)+CARRYIN)
      | RNDSIMPLE(PCIN>>17+(ACIN+D))
      | RNDSIMPLE(PCIN>>17+(ACIN+D)*(ACIN+D))
      | RNDSIMPLE(PCIN>>17+(ACIN+D)*(ACIN+D)+CARRYIN)
      | RNDSIMPLE(PCIN>>17+(ACIN+D)*B)
      | RNDSIMPLE(PCIN>>17+(ACIN+D)*B+CARRYIN)
      | RNDSIMPLE(PCIN>>17+(ACIN+D)*BCIN)
      | RNDSIMPLE(PCIN>>17+(ACIN+D)*BCIN+CARRYIN)
      | RNDSIMPLE(PCIN>>17+(ACIN+D)+CARRYIN)
      | RNDSIMPLE(PCIN>>17+(D+A))
      | RNDSIMPLE(PCIN>>17+(D+A)*(D+A))
      | RNDSIMPLE(PCIN>>17+(D+A)*(D+A)+CARRYIN)
      | RNDSIMPLE(PCIN>>17+(D+A)*B)
      | RNDSIMPLE(PCIN>>17+(D+A)*B+CARRYIN)
      | RNDSIMPLE(PCIN>>17+(D+A)*BCIN)
      | RNDSIMPLE(PCIN>>17+(D+A)*BCIN+CARRYIN)
      | RNDSIMPLE(PCIN>>17+(D+A)+CARRYIN)
      | RNDSIMPLE(PCIN>>17+(D+ACIN))
      | RNDSIMPLE(PCIN>>17+(D+ACIN)*(D+ACIN))
      | RNDSIMPLE(PCIN>>17+(D+ACIN)*(D+ACIN)+CARRYIN)
      | RNDSIMPLE(PCIN>>17+(D+ACIN)*B)
      | RNDSIMPLE(PCIN>>17+(D+ACIN)*B+CARRYIN)
      | RNDSIMPLE(PCIN>>17+(D+ACIN)*BCIN)
      | RNDSIMPLE(PCIN>>17+(D+ACIN)*BCIN+CARRYIN)
      | RNDSIMPLE(PCIN>>17+(D+ACIN)+CARRYIN)
      | RNDSIMPLE(PCIN>>17+(D-A))
      | RNDSIMPLE(PCIN>>17+(D-A)*(D-A))
      | RNDSIMPLE(PCIN>>17+(D-A)*(D-A)+CARRYIN)
      | RNDSIMPLE(PCIN>>17+(D-A)*B)
      | RNDSIMPLE(PCIN>>17+(D-A)*B+CARRYIN)
      | RNDSIMPLE(PCIN>>17+(D-A)*BCIN)
      | RNDSIMPLE(PCIN>>17+(D-A)*BCIN+CARRYIN)
      | RNDSIMPLE(PCIN>>17+(D-A)+CARRYIN)
      | RNDSIMPLE(PCIN>>17+(D-ACIN))
      | RNDSIMPLE(PCIN>>17+(D-ACIN)*(D-ACIN))
      | RNDSIMPLE(PCIN>>17+(D-ACIN)*(D-ACIN)+CARRYIN)
      | RNDSIMPLE(PCIN>>17+(D-ACIN)*B)
      | RNDSIMPLE(PCIN>>17+(D-ACIN)*B+CARRYIN)
      | RNDSIMPLE(PCIN>>17+(D-ACIN)*BCIN)
      | RNDSIMPLE(PCIN>>17+(D-ACIN)*BCIN+CARRYIN)
      | RNDSIMPLE(PCIN>>17+(D-ACIN)+CARRYIN)
      | RNDSIMPLE(PCIN>>17+A)
      | RNDSIMPLE(PCIN>>17+A*A)
      | RNDSIMPLE(PCIN>>17+A*A+CARRYIN)
      | RNDSIMPLE(PCIN>>17+A*B)
      | RNDSIMPLE(PCIN>>17+A*B+CARRYIN)
      | RNDSIMPLE(PCIN>>17+A*BCIN)
      | RNDSIMPLE(PCIN>>17+A*BCIN+CARRYIN)
      | RNDSIMPLE(PCIN>>17+A+CARRYIN)
      | RNDSIMPLE(PCIN>>17+ACIN)
      | RNDSIMPLE(PCIN>>17+ACIN*ACIN)
      | RNDSIMPLE(PCIN>>17+ACIN*ACIN+CARRYIN)
      | RNDSIMPLE(PCIN>>17+ACIN*B)
      | RNDSIMPLE(PCIN>>17+ACIN*B+CARRYIN)
      | RNDSIMPLE(PCIN>>17+ACIN*BCIN)
      | RNDSIMPLE(PCIN>>17+ACIN*BCIN+CARRYIN)
      | RNDSIMPLE(PCIN>>17+ACIN+CARRYIN)
      | RNDSIMPLE(PCIN>>17+B*(A+D))
      | RNDSIMPLE(PCIN>>17+B*(A+D)+CARRYIN)
      | RNDSIMPLE(PCIN>>17+B*(ACIN+D))
      | RNDSIMPLE(PCIN>>17+B*(ACIN+D)+CARRYIN)
      | RNDSIMPLE(PCIN>>17+B*(D+A))
      | RNDSIMPLE(PCIN>>17+B*(D+A)+CARRYIN)
      | RNDSIMPLE(PCIN>>17+B*(D+ACIN))
      | RNDSIMPLE(PCIN>>17+B*(D+ACIN)+CARRYIN)
      | RNDSIMPLE(PCIN>>17+B*(D-A))
      | RNDSIMPLE(PCIN>>17+B*(D-A)+CARRYIN)
      | RNDSIMPLE(PCIN>>17+B*(D-ACIN))
      | RNDSIMPLE(PCIN>>17+B*(D-ACIN)+CARRYIN)
      | RNDSIMPLE(PCIN>>17+B*A)
      | RNDSIMPLE(PCIN>>17+B*A+CARRYIN)
      | RNDSIMPLE(PCIN>>17+B*ACIN)
      | RNDSIMPLE(PCIN>>17+B*ACIN+CARRYIN)
      | RNDSIMPLE(PCIN>>17+B*D)
      | RNDSIMPLE(PCIN>>17+B*D+CARRYIN)
      | RNDSIMPLE(PCIN>>17+BCIN*(A+D))
      | RNDSIMPLE(PCIN>>17+BCIN*(A+D)+CARRYIN)
      | RNDSIMPLE(PCIN>>17+BCIN*(ACIN+D))
      | RNDSIMPLE(PCIN>>17+BCIN*(ACIN+D)+CARRYIN)
      | RNDSIMPLE(PCIN>>17+BCIN*(D+A))
      | RNDSIMPLE(PCIN>>17+BCIN*(D+A)+CARRYIN)
      | RNDSIMPLE(PCIN>>17+BCIN*(D+ACIN))
      | RNDSIMPLE(PCIN>>17+BCIN*(D+ACIN)+CARRYIN)
      | RNDSIMPLE(PCIN>>17+BCIN*(D-A))
      | RNDSIMPLE(PCIN>>17+BCIN*(D-A)+CARRYIN)
      | RNDSIMPLE(PCIN>>17+BCIN*(D-ACIN))
      | RNDSIMPLE(PCIN>>17+BCIN*(D-ACIN)+CARRYIN)
      | RNDSIMPLE(PCIN>>17+BCIN*A)
      | RNDSIMPLE(PCIN>>17+BCIN*A+CARRYIN)
      | RNDSIMPLE(PCIN>>17+BCIN*ACIN)
      | RNDSIMPLE(PCIN>>17+BCIN*ACIN+CARRYIN)
      | RNDSIMPLE(PCIN>>17+BCIN*D)
      | RNDSIMPLE(PCIN>>17+BCIN*D+CARRYIN)
      | RNDSIMPLE(PCIN>>17+C)
      | RNDSIMPLE(PCIN>>17+C+CARRYIN)
      | RNDSIMPLE(PCIN>>17+CARRYIN)
      | RNDSIMPLE(PCIN>>17+CONCAT)
      | RNDSIMPLE(PCIN>>17+CONCAT+C)
      | RNDSIMPLE(PCIN>>17+CONCAT+C+CARRYIN)
      | RNDSIMPLE(PCIN>>17+CONCAT+CARRYIN)
      | RNDSIMPLE(PCIN>>17+D)
      | RNDSIMPLE(PCIN>>17+D*B)
      | RNDSIMPLE(PCIN>>17+D*B+CARRYIN)
      | RNDSIMPLE(PCIN>>17+D*BCIN)
      | RNDSIMPLE(PCIN>>17+D*BCIN+CARRYIN)
      | RNDSIMPLE(PCIN>>17+D*D)
      | RNDSIMPLE(PCIN>>17+D*D+CARRYIN)
      | RNDSIMPLE(PCIN>>17+D+CARRYIN)
      | RNDSIMPLE(PCIN>>17+P)
      | RNDSIMPLE(PCIN>>17+P+C)
      | RNDSIMPLE(PCIN>>17+P+C+CARRYIN)
      | RNDSIMPLE(PCIN>>17+P+CARRYIN)
      | RNDSYM((A+D))
      | RNDSYM((A+D)*(A+D))
      | RNDSYM((A+D)*B)
      | RNDSYM((A+D)*BCIN)
      | RNDSYM((ACIN+D))
      | RNDSYM((ACIN+D)*(ACIN+D))
      | RNDSYM((ACIN+D)*B)
      | RNDSYM((ACIN+D)*BCIN)
      | RNDSYM((D+A))
      | RNDSYM((D+A)*(D+A))
      | RNDSYM((D+A)*B)
      | RNDSYM((D+A)*BCIN)
      | RNDSYM((D+ACIN))
      | RNDSYM((D+ACIN)*(D+ACIN))
      | RNDSYM((D+ACIN)*B)
      | RNDSYM((D+ACIN)*BCIN)
      | RNDSYM((D-A))
      | RNDSYM((D-A)*(D-A))
      | RNDSYM((D-A)*B)
      | RNDSYM((D-A)*BCIN)
      | RNDSYM((D-ACIN))
      | RNDSYM((D-ACIN)*(D-ACIN))
      | RNDSYM((D-ACIN)*B)
      | RNDSYM((D-ACIN)*BCIN)
      | RNDSYM(A)
      | RNDSYM(A*A)
      | RNDSYM(A*B)
      | RNDSYM(A*BCIN)
      | RNDSYM(ACIN)
      | RNDSYM(ACIN*ACIN)
      | RNDSYM(ACIN*B)
      | RNDSYM(ACIN*BCIN)
      | RNDSYM(B*(A+D))
      | RNDSYM(B*(ACIN+D))
      | RNDSYM(B*(D+A))
      | RNDSYM(B*(D+ACIN))
      | RNDSYM(B*(D-A))
      | RNDSYM(B*(D-ACIN))
      | RNDSYM(B*A)
      | RNDSYM(B*ACIN)
      | RNDSYM(B*D)
      | RNDSYM(BCIN*(A+D))
      | RNDSYM(BCIN*(ACIN+D))
      | RNDSYM(BCIN*(D+A))
      | RNDSYM(BCIN*(D+ACIN))
      | RNDSYM(BCIN*(D-A))
      | RNDSYM(BCIN*(D-ACIN))
      | RNDSYM(BCIN*A)
      | RNDSYM(BCIN*ACIN)
      | RNDSYM(BCIN*D)
      | RNDSYM(D)
      | RNDSYM(D*B)
      | RNDSYM(D*BCIN)
      | RNDSYM(D*D)
      | RNDSYM(P)
      | RNDSYM(PCIN)
      settings: infoedit=<html>Valid operands: CONCAT, P, C, PCIN, P>>17, PCIN>>17, CARRYIN, CARRYCASCIN, ACIN, A, BCIN,B<br><br>Valid operators: +, -, *, ()<br><br>Valid functions: RNDSIMPLE, RNDSYM<br><br>Instructions are case insensitive and tolerate spaces.</html>
  Reinterpret : xbsIndex_r4/Reinterpret  force_arith_type=on  arith_type="Signed  (2's comp)"  force_bin_pt=on  bin_pt=coeff_frac_width  block_type=reinterpret
      settings: infoedit="Changes signal type without altering the binary representation.   You can changed the signal between signed and unsigned, and relocate the binary point.<P><P>Hardware notes: In hardware this block costs nothing.<P><P>Example:  Suppose the input is 6 bits wide, signed, with 2 fractional bits, and the output is forced to unsigned with 0 fractional bits.  Then an input of -2.0 (1110.00 in binary 2's complement) becomes an output of 56 (111000 in binary)."  block_version=9.1.01
  Slice : xbsIndex_r4/Slice  nbits=coeff_width  boolean_output=off  mode=Lower Bit Location + Width  bit1=0  base1=MSB of Input  bit0=0  base0=LSB of Input  dbl_ovrd=off  block_type=slice
      settings: infoedit="Extracts a given range of bits from each input sample and presents it at the output.  The output type is ordinarily unsigned with binary point at zero, but can be Boolean when the slice is one bit wide.<P><P>Hardware notes: In hardware this block costs nothing."  block_version=9.1.01
  Slice1 : xbsIndex_r4/Slice  nbits=0  boolean_output=off  mode=Two Bit Locations  bit1=0  base1=MSB of Input  bit0=coeff_width  base0=LSB of Input  dbl_ovrd=off  block_type=slice
      settings: infoedit="Extracts a given range of bits from each input sample and presents it at the output.  The output type is ordinarily unsigned with binary point at zero, but can be Boolean when the slice is one bit wide.<P><P>Hardware notes: In hardware this block costs nothing."  block_version=9.1.01
  c_to_ri : casper_library_misc/c_to_ri  n_bits=data_width  bin_pt=data_width-1
      settings: SourceProductName=CASPER DSP Blockset
  c_to_ri1 : casper_library_misc/c_to_ri  n_bits=36+3  bin_pt=34
      settings: AttributesFormatString=39_34 r/i  SourceProductName=CASPER DSP Blockset
  delay_bram : Subsystem  AncestorBlock=casper_library_delays/delay_bram  mask(delay_bram){DelayLen=delay+1, bram_latency=bram_latency, use_dsp48=off, async=off}
      mask prompts: DelayLen "Delay By:"; bram_latency "BRAM Latency"; use_dsp48 "Use DSP48"; async "asynchronous operation"
      mask help: A delay block that uses BRAM for its storage.
      mask init:
      | delay_bram_init(gcb, ...
      |     'DelayLen', DelayLen, ...
      |     'bram_latency', bram_latency, ...
      |     'use_dsp48', use_dsp48, ...
      |     'async', async);
      mask Help: eval('xlWeb(''http://casper.berkeley.edu/wiki/Delay_bram'')')
  ri_to_c : Subsystem
  sync_delay : casper_library_delays/sync_delay  DelayLen=delay+1
      settings: SourceProductName=CASPER DSP Blockset
  dout : Outport  VectorParamsAs1DForOutWhenUnconnected=off
  sync_out : Outport  Port=2  VectorParamsAs1DForOutWhenUnconnected=off
  coeff_out : Outport  Port=3  VectorParamsAs1DForOutWhenUnconnected=off
  taps_out : Outport  Port=4  VectorParamsAs1DForOutWhenUnconnected=off
  wires:
  Convert1/1 -> ri_to_c/2(im)
  Convert/1 -> ri_to_c/1(re)
  DSP Macro 1.1/1 -> Convert1/1
  DSP Macro 1.0/1 -> Convert/1
  c_to_ri1/2 -> DSP Macro 1.1/3
  c_to_ri1/1 -> DSP Macro 1.0/3
  taps/1 -> c_to_ri1/1
  Reinterpret/1 -> DSP Macro 1.0/2, DSP Macro 1.1/2
  Slice/1 -> Reinterpret/1
  Slice1/1 -> coeff_out/1
  coeff/1 -> Slice1/1, Slice/1
  ri_to_c/1(c) -> taps_out/1
  sync_delay/1 -> sync_out/1
  sync/1 -> sync_delay/1
  c_to_ri/1 -> DSP Macro 1.0/1
  c_to_ri/2 -> DSP Macro 1.1/1
  din/1 -> delay_bram/1(din), c_to_ri/1
  delay_bram/1(dout) -> dout/1
